simulink model "MCVT_Integration"  (R2020a)
settings: Solver=ode8  StartTime=0.0  StopTime=T_end  FixedStep=dt  RelTol=1e-3  EnableMultiTasking=on
values from neighbouring files (symbols this model uses):
  assumed_power_voltage = 1.25e2    (= 125)    [MCVT_Input_File.m]    (also in MCVT_Run_QuickCheck.m)
  battery_power_toggle = 1    [MCVT_Input_File.m]    (also in MCVT_Run_QuickCheck.m)
  battery_trickle_charge_param = 0.1    [MCVT_Input_File.m]    (also in MCVT_Run_QuickCheck.m)
  battery_trickle_leakage_param = 0.01    [MCVT_Input_File.m]    (also in MCVT_Run_QuickCheck.m)
  damage_case_id = 3    [MCVT_Input_File.m]    (also in MCVT_Run_QuickCheck.m = 1)
  dt = 1/fs    (= 0.001)    [MCVT_Run_QuickCheck.m]    (also in MCVT_Sim_Set.m)
  dt_2 = 1/fs_2    (= 0.001)    [MCVT_Run_QuickCheck.m]    (also in MCVT_Sim_Set.m)
  dt_3 = 1/fs_3    (= 0.001)    [MCVT_Run_QuickCheck.m]    (also in MCVT_Sim_Set.m)
  dt_5 = 1/fs_5    (= 0.005)    [MCVT_Run_QuickCheck.m]    (also in MCVT_Sim_Set.m)
  dt_8 = 1/fs_8    (= 0.05)    [MCVT_Run_QuickCheck.m]    (also in MCVT_Sim_Set.m)
  dt_9 = 1/fs_9    (= 0.001)    [MCVT_Run_QuickCheck.m]    (also in MCVT_Sim_Set.m)
  dust_met_duration = 3    [MCVT_Input_File.m]    (also in MCVT_Run_QuickCheck.m = 2)
  ECLSS_indoor_set_temperature = 298.15    [MCVT_Input_File.m]    (also in MCVT_Run_QuickCheck.m)
  energy_store_max_o = 150    [MCVT_Input_File.m]    (also in MCVT_Run_QuickCheck.m)
  energy_store_o = 120    [MCVT_Input_File.m]    (also in MCVT_Run_QuickCheck.m)
  fs = 1000    [MCVT_Run_QuickCheck.m]    (also in MCVT_Sim_Set.m)
  gain_charge_param = 1    [MCVT_Input_File.m]    (also in MCVT_Run_QuickCheck.m)
  ground_temperature = 373    [MCVT_Habitat_Design_Input_File.m]    (also in MCVT_Run_QuickCheck.m)
  I_max_charge = 800    [MCVT_Input_File.m]    (also in MCVT_Run_QuickCheck.m)
  I_max_discharge = 800    [MCVT_Input_File.m]    (also in MCVT_Run_QuickCheck.m)
  initial_pressure = 1e5    (= 100000)    [MCVT_Input_File.m]    (also in MCVT_Run_QuickCheck.m)
  initial_temperature = 295    [MCVT_Input_File.m]    (also in MCVT_Run_QuickCheck.m)
  interior_leak_rate = 0.00415    [MCVT_Input_File.m]    (also in MCVT_Run_QuickCheck.m)
  launch_dust_accum_start_time = 10    [MCVT_Input_File.m]    (also in MCVT_Run_QuickCheck.m = 2)
  launch_dust_accum_stop_time = 12    [MCVT_Input_File.m]    (also in MCVT_Run_QuickCheck.m = 3)
  meteorite_impact_time = 2    [MCVT_Input_File.m]    (also in MCVT_Run_QuickCheck.m = 1)
  nuclear_power_toggle = 1    [MCVT_Input_File.m]    (also in MCVT_Run_QuickCheck.m)
  print_interval = 30    [MCVT_Input_File.m]
  SOC_lower_thres = 0.5    [MCVT_Input_File.m]    (also in MCVT_Run_QuickCheck.m)
  SOC_upper_thres = 0.9    [MCVT_Input_File.m]    (also in MCVT_Run_QuickCheck.m)
  solar_power_toggle = 1    [MCVT_Input_File.m]    (also in MCVT_Run_QuickCheck.m)
  space_temperature = 4    [MCVT_Habitat_Design_Input_File.m]    (also in MCVT_Run_QuickCheck.m)
  Start_angle = 90    [MCVT_Input_File.m]    (also in MCVT_Run_QuickCheck.m)
undefined symbols (not defined in the file or in its folder): A_flow_restrictor, ACTIVITY_LOOKUP_TABLE, air, Aref, Bmap, Bmap2, Bmap3, comms, DiscIntNeverNeededParam, DmgSPL, dust_parameters, ECLSS_dust_damage_repair_time, ECLSS_heater_capacity, ECLSS_IPS_restrictor, ECLSS_IPS_setpointPressure, ECLSS_paint_damage_repair_time, ECLSS_radiatorLoop_discreteNumber, ECLSS_Vst, emptyActivity, expected_heat_add, FI, habitat, HIEM2SMM, HIEM2STM, HIEMDamageElements, impact_numbering, initial_SPLT_damage_index, input_air, input_Cond, input_CP, input_discretization, input_EV, input_Evap, input_radiatorLoop, K_ECLSS_dust_single, K_ECLSS_paint_single, K_Structure_damage_single, Kref, kW_to_W, meteoroidDustDep, mFRF, ndelays, nominalDustDep, noverlap, nuclear_toggle_gain, output_limits, panel_paint0, Ps0, reference_temperature, RepairTime_ECLSS_factor, Sensor_Location_SMM, SMM_T0, SMM_T1, SMM_T2, SMM_T3, SMM_T4, SMM_T4_0, SMM_T4_1, SMM_T4_2, solar_FDD_K ... (+73)
libraries: neural x350, simulink x39, xpcutilitieslib x7, xpcrtudplib x7, dspstat3 x1
(part 35 of 43)

== system MCVT_Integration/Sys2 - Structural System/Sys2_Structural_System/Fault Detection and Diagnosis (FDD) with Binary Classifer/Subsystem/Enabled Subsystem3/Pattern Recognition Neural Network1/Layer 3/Delays 1 ==
  "p{3}" : Inport  PortDimensions=20  SampleTime=0.001
      mask Display: e = 0.06;c1 = [120 230 180]/255;c2 = ([120 230 180]+0)/(256+128);c3 = ([120 230 180]+128)/(256+128);patch([0 1 1 0],[0 0 1 1],c1);patch([0 1 1 1-e 1-e 0+e],[0 0 1 1-e 0+e 0+e],c2);patch([0 0 1 1-e 0+e 0+e],[0 1 1 1-e 1-e 0+e],c3);plot([0 1 1 0 0],[0 0 1 1 0]);
  mux : Mux  Inputs=1
      mask Display: e = 0.06;c1 = [120 150 230]/255;c2 = ([120 150 230]+0)/383;c3 = ([120 150 230]+128)/383;patch([0 1 1 0],[0 0 1 1],c1);patch([0 1 1 1-e 1-e 0+e],[0 0 1 1-e 0+e 0+e],c2);patch([0 0 1 1-e 0+e 0+e],[0 1 1 1-e 1-e 0+e],c3);plot([0 1 1 0 0],[0 0 1 1 0]);disp('Mux')
  "pd{3,2}" : Outport
      mask Display: e = 0.06;c1 = [120 230 180]/255;c2 = ([120 230 180]+0)/(256+128);c3 = ([120 230 180]+128)/(256+128);patch([0 1 1 0],[0 0 1 1],c1);patch([0 1 1 1-e 1-e 0+e],[0 0 1 1-e 0+e 0+e],c2);patch([0 0 1 1-e 0+e 0+e],[0 1 1 1-e 1-e 0+e],c3);plot([0 1 1 0 0],[0 0 1 1 0]);
  wires:
  "p{3}"/1 -> mux/1
  mux/1 -> "pd{3,2}"/1

== system MCVT_Integration/Sys2 - Structural System/Sys2_Structural_System/Fault Detection and Diagnosis (FDD) with Binary Classifer/Subsystem/Enabled Subsystem3/Pattern Recognition Neural Network1/Layer 3/LW{3,2} ==
  "ad{3,2}" : Inport  PortDimensions=20  SampleTime=0.001
      mask Display: e = 0.06;c1 = [120 230 180]/255;c2 = ([120 230 180]+0)/(256+128);c3 = ([120 230 180]+128)/(256+128);patch([0 1 1 0],[0 0 1 1],c1);patch([0 1 1 1-e 1-e 0+e],[0 0 1 1-e 0+e 0+e],c2);patch([0 0 1 1-e 0+e 0+e],[0 1 1 1-e 1-e 0+e],c3);plot([0 1 1 0 0],[0 0 1 1 0]);
  "IW{3,2}(1,:)'" : Constant  Value=[-0.11418317317423039292112463272133027203381061553955078125;0.263496798393575704100300072241225279867649078369140625;0.312934394505613722170522805754444561898708343505859375;-0.450418381981469717612043268673005513846874237060546875;0.008234437834952347545769413272864767350256443023681640625;-0.462923222718843174749991931093973107635974884033203125;0.5964544880257796055644803345785476267337799072265625;-0.4783472265888535357447608475922606885433197021484375;-0.09036306949382867659270601734533556737005710601806640625;0.12308048153058916140967227192959398962557315826416015625;0.10743009166485244232358553517769905738532543182373046875;-0.481697721023931324513256413410999812185764312744140625;-0.47876976535250570510271472812746651470661163330078125;-0.711626168608504361401401183684356510639190673828125;0.225177975554882225939223872046568430960178375244140625;0.681899131620984366719540048507042229175567626953125;0.460863606080788301699868725336273200809955596923828125;-0.323125050863476370732740861058118753135204315185546875;-0.25662906632515347649103887306409887969493865966796875;0.480838665710967172461920426940196193754673004150390625]
      mask Display: e = 0.06;c1 = [120 150 230]/255;c2 = ([120 150 230]+0)/383;c3 = ([120 150 230]+128)/383;patch([0 1 1 0],[0 0 1 1],c1);patch([0 1 1 1-e 1-e 0+e],[0 0 1 1-e 0+e 0+e],c2);patch([0 0 1 1-e 0+e 0+e],[0 1 1 1-e 1-e 0+e],c3);plot([0 1 1 0 0],[0 0 1 1 0]);disp('weights');
  "IW{3,2}(10,:)'" : Constant  Value=[0.3861400843114013436974119031219743192195892333984375;0.3125216652004194628489130991511046886444091796875;-0.595476598820065650130572976195253431797027587890625;-0.037119852898883122505058196338723064400255680084228515625;0.189111040823012388489843260686029680073261260986328125;0.420303286434084044653758382992236874997615814208984375;-0.2676880868573281446742839762009680271148681640625;0.1753980171191553971699050862298463471233844757080078125;0.39560842290195019632648154583876021206378936767578125;0.69829375106497781899861365673132240772247314453125;0.50404441039708292038312720251269638538360595703125;0.252775726492291241687127012482960708439350128173828125;-0.1450315262218064005228512769463122822344303131103515625;0.0859287412860451904617065110869589261710643768310546875;0.133941298854357959680072553965146653354167938232421875;0.22618240937400890810948794751311652362346649169921875;0.379638748312004725704582597245462238788604736328125;-0.21496011361299871555985419036005623638629913330078125;-0.306329634482241985882211565694888122379779815673828125;0.302106512168412055796551385355996899306774139404296875]
      mask Display: e = 0.06;c1 = [120 150 230]/255;c2 = ([120 150 230]+0)/383;c3 = ([120 150 230]+128)/383;patch([0 1 1 0],[0 0 1 1],c1);patch([0 1 1 1-e 1-e 0+e],[0 0 1 1-e 0+e 0+e],c2);patch([0 0 1 1-e 0+e 0+e],[0 1 1 1-e 1-e 0+e],c3);plot([0 1 1 0 0],[0 0 1 1 0]);disp('weights');
  "IW{3,2}(2,:)'" : Constant  Value=[-0.163390928991248796098290085865301080048084259033203125;-0.0951760108873469234946895767279784195125102996826171875;-0.84593655133122869216322214924730360507965087890625;0.46899503026038946718045963280019350349903106689453125;-0.8490909409678917274533205272746272385120391845703125;-0.57545479794395382189264864791766740381717681884765625;-0.324175505751264803055988750202232040464878082275390625;0.364041861382856046791545168161974288523197174072265625;-0.2544481589174762969918219823739491403102874755859375;0.28990490623402187519985773178632371127605438232421875;-0.08466602028875479246661228671655408106744289398193359375;0.70073781097509135751266740044229663908481597900390625;-0.41615489274903116889703369452035985887050628662109375;0.2424679765837617073653831312185502611100673675537109375;-0.7464754937890354380414237311924807727336883544921875;-0.1596535806179091299128458558698184788227081298828125;0.7130628957660773981075408300966955721378326416015625;-0.43840295015644847875790901525761000812053680419921875;0.284476216686578020631515073546324856579303741455078125;0.447583082598702441945448526894324459135532379150390625]
      mask Display: e = 0.06;c1 = [120 150 230]/255;c2 = ([120 150 230]+0)/383;c3 = ([120 150 230]+128)/383;patch([0 1 1 0],[0 0 1 1],c1);patch([0 1 1 1-e 1-e 0+e],[0 0 1 1-e 0+e 0+e],c2);patch([0 0 1 1-e 0+e 0+e],[0 1 1 1-e 1-e 0+e],c3);plot([0 1 1 0 0],[0 0 1 1 0]);disp('weights');
  "IW{3,2}(3,:)'" : Constant  Value=[0.0098602709082808159823940741262049414217472076416015625;-0.40815757787930284639088540643570013344287872314453125;0.12336924569621048053402745381390559487044811248779296875;-0.6190044151957840856681514196679927408695220947265625;-0.564487784933820790911340736784040927886962890625;0.54853651299544214925418827988323755562305450439453125;-1.258259749472828925576095571159385144710540771484375;-0.77927996917740427473830777671537362039089202880859375;0.16813033094256812916000853874720633029937744140625;0.10729529541650513480011142064540763385593891143798828125;0.262661701867050567660299975614179857075214385986328125;-0.0733259301165432308966529717508819885551929473876953125;1.22466710827414804185764296562410891056060791015625;0.271802237285291459212288600610918365418910980224609375;-0.71351834644215295089253459082101471722126007080078125;-0.74660981018017091859206857407116331160068511962890625;0.5983682870257907016053877669037319719791412353515625;-0.01351833734841678680282939239987172186374664306640625;0.051967795942245952056648405914529575966298580169677734375;0.1434699887749444624507333401197683997452259063720703125]
      mask Display: e = 0.06;c1 = [120 150 230]/255;c2 = ([120 150 230]+0)/383;c3 = ([120 150 230]+128)/383;patch([0 1 1 0],[0 0 1 1],c1);patch([0 1 1 1-e 1-e 0+e],[0 0 1 1-e 0+e 0+e],c2);patch([0 0 1 1-e 0+e 0+e],[0 1 1 1-e 1-e 0+e],c3);plot([0 1 1 0 0],[0 0 1 1 0]);disp('weights');
  "IW{3,2}(4,:)'" : Constant  Value=[-0.039394049345288499608397358997535775415599346160888671875;-0.320240693032457890421227375554735772311687469482421875;0.203468281254232497001765977984177879989147186279296875;0.138427932331368575002983334343298338353633880615234375;0.488490423588747113559094259471748955547809600830078125;0.276252854526421176561967740781255997717380523681640625;0.104006018020532609025252668288885615766048431396484375;0.51873376787390912401320974822738207876682281494140625;0.48405322090183655614481494922074489295482635498046875;-0.06304979425881369892170624780192156322300434112548828125;-0.392143975943062106370717856407281942665576934814453125;-0.75218739963867531361785268018138594925403594970703125;0.71739453453784574055163147932034917175769805908203125;-0.443935510913416486733495958105777390301227569580078125;0.78728550848033951314874911986407823860645294189453125;-0.120688688580087466473145241252495907247066497802734375;-0.53856777060676896962121418255264870822429656982421875;0.3444710945141284952342175529338419437408447265625;0.25306929741619488272164062436786480247974395751953125;0.331976039764520780561696255972492508590221405029296875]
      mask Display: e = 0.06;c1 = [120 150 230]/255;c2 = ([120 150 230]+0)/383;c3 = ([120 150 230]+128)/383;patch([0 1 1 0],[0 0 1 1],c1);patch([0 1 1 1-e 1-e 0+e],[0 0 1 1-e 0+e 0+e],c2);patch([0 0 1 1-e 0+e 0+e],[0 1 1 1-e 1-e 0+e],c3);plot([0 1 1 0 0],[0 0 1 1 0]);disp('weights');
  "IW{3,2}(5,:)'" : Constant  Value=[0.323360971356433768431060116199660114943981170654296875;-0.281984417075820503750804846276878379285335540771484375;-0.289282489355113858753298927695141173899173736572265625;0.4782575856980688655539779574610292911529541015625;-0.642831136730397378897805538144893944263458251953125;1.0145734120541420875127869294374249875545501708984375;0.2595396344103828578653292424860410392284393310546875;0.66796656307939361596481830929405987262725830078125;-0.46150839887887240564623425598256289958953857421875;0.306587228188199223399834636438754387199878692626953125;-0.94949409796797734628626130870543420314788818359375;-0.03140176831624992559444109474497963674366474151611328125;0.295677475903499298315324494978995062410831451416015625;-0.5493980409988299395962485505151562392711639404296875;0.403008193931628400097366693444200791418552398681640625;0.9733051449238032670763232090394012629985809326171875;-0.8510176648196960957903911548783071339130401611328125;-0.2207833887391404126265825880182092078030109405517578125;-0.278018396739915463200532030896283686161041259765625;0.393882424770033490180054513984941877424716949462890625]
      mask Display: e = 0.06;c1 = [120 150 230]/255;c2 = ([120 150 230]+0)/383;c3 = ([120 150 230]+128)/383;patch([0 1 1 0],[0 0 1 1],c1);patch([0 1 1 1-e 1-e 0+e],[0 0 1 1-e 0+e 0+e],c2);patch([0 0 1 1-e 0+e 0+e],[0 1 1 1-e 1-e 0+e],c3);plot([0 1 1 0 0],[0 0 1 1 0]);disp('weights');
  "IW{3,2}(6,:)'" : Constant  Value=[-0.3606616604959353988846260108402930200099945068359375;-0.1704191509480204036464101591263897716999053955078125;0.150526929813042997796657118669827468693256378173828125;0.1472315563777921998411812865015235729515552520751953125;-0.1278164823918129522350994875523610971868038177490234375;-1.046809064148225498769306796020828187465667724609375;0.582795720158978181046904865070246160030364990234375;0.75223224770795427129854715531109832227230072021484375;0.33523115012585902316999408867559395730495452880859375;-0.338211234277506933576518122208653949201107025146484375;-0.390605230615673482130745242102420888841152191162109375;-0.287165688343693215056617873415234498679637908935546875;-0.70909893212667751694056050837389193475246429443359375;-0.7038433128654613124552952285739593207836151123046875;-0.86812992371547170744605637082713656127452850341796875;-0.1746870548125196986877227800505352206528186798095703125;-0.410264069210815929267255341983400285243988037109375;-0.48871178689720362608994719266775064170360565185546875;-0.05532824759820657856135284191623213700950145721435546875;0.05704494121705543097089474713357049040496349334716796875]
      mask Display: e = 0.06;c1 = [120 150 230]/255;c2 = ([120 150 230]+0)/383;c3 = ([120 150 230]+128)/383;patch([0 1 1 0],[0 0 1 1],c1);patch([0 1 1 1-e 1-e 0+e],[0 0 1 1-e 0+e 0+e],c2);patch([0 0 1 1-e 0+e 0+e],[0 1 1 1-e 1-e 0+e],c3);plot([0 1 1 0 0],[0 0 1 1 0]);disp('weights');
  "IW{3,2}(7,:)'" : Constant  Value=[0.11984386036742382397601858201596769504249095916748046875;0.347080609380766791360883871675468981266021728515625;0.0917862807948270631452913903558510355651378631591796875;-0.288490135071363951180245521754841320216655731201171875;0.63114059686687029593343822853057645261287689208984375;-0.5584052675142625599136181335779838263988494873046875;0.53125277720215191568087220730376429855823516845703125;-0.3716338061699360206802111861179582774639129638671875;-0.05721538948189093531926374680551816709339618682861328125;-0.71575407318186801131076890669646672904491424560546875;-0.08369009494839028950874393331105238758027553558349609375;-0.215724270354954394957047725256416015326976776123046875;1.2396797863463160371821913940948434174060821533203125;0.47994944326806088241710313013754785060882568359375;-0.00717360076498131486733011996648201602511107921600341796875;0.22905992881372438940701385945430956780910491943359375;-0.976685274095676181360659029451198875904083251953125;-0.180881724828344025990389809521730057895183563232421875;-0.294597694843611368487046320296940393745899200439453125;0.04199206334362116932457098528175265528261661529541015625]
      mask Display: e = 0.06;c1 = [120 150 230]/255;c2 = ([120 150 230]+0)/383;c3 = ([120 150 230]+128)/383;patch([0 1 1 0],[0 0 1 1],c1);patch([0 1 1 1-e 1-e 0+e],[0 0 1 1-e 0+e 0+e],c2);patch([0 0 1 1-e 0+e 0+e],[0 1 1 1-e 1-e 0+e],c3);plot([0 1 1 0 0],[0 0 1 1 0]);disp('weights');
  "IW{3,2}(8,:)'" : Constant  Value=[-0.21342261365704862097203431403613649308681488037109375;0.35624143718627421240086050602258183062076568603515625;0.326618800376075990588020658833556808531284332275390625;0.06597532960682862823720284950468339957296848297119140625;-0.17461288648652029120711404175381176173686981201171875;-0.263005374932626601047758185814018361270427703857421875;-0.049151473469004801353232636529355659149587154388427734375;0.766371952396857292200138545013032853603363037109375;-0.1355212253420195744890719424802227877080440521240234375;-0.56439442500416614478808696730993688106536865234375;-0.71372482706963469301086888663121499121189117431640625;0.12115721620020099280612413394919713027775287628173828125;-0.254628175010240986875231783415074460208415985107421875;0.2614167249770866785496536977007053792476654052734375;0.57295880892063089095955774610047228634357452392578125;0.54430137291944247213137941798777319490909576416015625;0.59699489786736725793758751024142839014530181884765625;-0.085575093688173564654420033548376522958278656005859375;0.038297841824984357739225515615544281899929046630859375;0.372723897650229674116673095340956933796405792236328125]
      mask Display: e = 0.06;c1 = [120 150 230]/255;c2 = ([120 150 230]+0)/383;c3 = ([120 150 230]+128)/383;patch([0 1 1 0],[0 0 1 1],c1);patch([0 1 1 1-e 1-e 0+e],[0 0 1 1-e 0+e 0+e],c2);patch([0 0 1 1-e 0+e 0+e],[0 1 1 1-e 1-e 0+e],c3);plot([0 1 1 0 0],[0 0 1 1 0]);disp('weights');
  "IW{3,2}(9,:)'" : Constant  Value=[-0.1914423406104446556685871883018990047276020050048828125;-0.393308930523766420161990708947996608912944793701171875;1.2635378199134894572353005059994757175445556640625;1.059262594219074760104604138177819550037384033203125;-0.73547758546770169996165122938691638410091400146484375;-1.7230018270653120193713903063326142728328704833984375;0.193088656561395455302232448957511223852634429931640625;-0.1291887260637646084315832695210701785981655120849609375;0.08111166291770897929236383561146794818341732025146484375;0.07141734494336980654249913413877948187291622161865234375;0.93639924412624753191636273186304606497287750244140625;0.369705966288347009918169305819901637732982635498046875;-0.5856153767568719192837534137652255594730377197265625;-0.449137029161876977223499807223561219871044158935546875;0.65634022147763559917876818872173316776752471923828125;0.68121028217221801792646829198929481208324432373046875;1.0442129309195211650518331225612200796604156494140625;-0.531155452284312712407654544222168624401092529296875;0.435977640337065930342674846542649902403354644775390625;0.7223516834459691171588247016188688576221466064453125]
      mask Display: e = 0.06;c1 = [120 150 230]/255;c2 = ([120 150 230]+0)/383;c3 = ([120 150 230]+128)/383;patch([0 1 1 0],[0 0 1 1],c1);patch([0 1 1 1-e 1-e 0+e],[0 0 1 1-e 0+e 0+e],c2);patch([0 0 1 1-e 0+e 0+e],[0 1 1 1-e 1-e 0+e],c3);plot([0 1 1 0 0],[0 0 1 1 0]);disp('weights');
  Mux : Mux  Inputs=10
      mask Display: e = 0.06;c1 = [120 150 230]/255;c2 = ([120 150 230]+0)/383;c3 = ([120 150 230]+128)/383;patch([0 1 1 0],[0 0 1 1],c1);patch([0 1 1 1-e 1-e 0+e],[0 0 1 1-e 0+e 0+e],c2);patch([0 0 1 1-e 0+e 0+e],[0 1 1 1-e 1-e 0+e],c3);plot([0 1 1 0 0],[0 0 1 1 0]);disp('Mux');
  dotprod{1..10} (10 alike) : neural/Weight Functions/dotprod
  "lz{3,2}" : Outport
      mask Display: e = 0.06;c1 = [120 230 180]/255;c2 = ([120 230 180]+0)/(256+128);c3 = ([120 230 180]+128)/(256+128);patch([0 1 1 0],[0 0 1 1],c1);patch([0 1 1 1-e 1-e 0+e],[0 0 1 1-e 0+e 0+e],c2);patch([0 0 1 1-e 0+e 0+e],[0 1 1 1-e 1-e 0+e],c3);plot([0 1 1 0 0],[0 0 1 1 0]);
  wires:
  "IW{3,2}(1,:)'"/1 -> dotprod1/1
  "ad{3,2}"/1 -> dotprod1/2, dotprod2/2, dotprod3/2, dotprod4/2, dotprod5/2, dotprod6/2, dotprod7/2, dotprod8/2, dotprod9/2, dotprod10/2
  dotprod1/1 -> Mux/1
  "IW{3,2}(2,:)'"/1 -> dotprod2/1
  dotprod2/1 -> Mux/2
  "IW{3,2}(3,:)'"/1 -> dotprod3/1
  dotprod3/1 -> Mux/3
  "IW{3,2}(4,:)'"/1 -> dotprod4/1
  dotprod4/1 -> Mux/4
  "IW{3,2}(5,:)'"/1 -> dotprod5/1
  dotprod5/1 -> Mux/5
  "IW{3,2}(6,:)'"/1 -> dotprod6/1
  dotprod6/1 -> Mux/6
  "IW{3,2}(7,:)'"/1 -> dotprod7/1
  dotprod7/1 -> Mux/7
  "IW{3,2}(8,:)'"/1 -> dotprod8/1
  dotprod8/1 -> Mux/8
  "IW{3,2}(9,:)'"/1 -> dotprod9/1
  dotprod9/1 -> Mux/9
  "IW{3,2}(10,:)'"/1 -> dotprod10/1
  dotprod10/1 -> Mux/10
  Mux/1 -> "lz{3,2}"/1

== system MCVT_Integration/Sys2 - Structural System/Sys2_Structural_System/Fault Detection and Diagnosis (FDD) with Binary Classifer/Subsystem/Enabled Subsystem3/Pattern Recognition Neural Network1/Layer 4/Delays 1 ==
  "p{4}" : Inport  PortDimensions=10  SampleTime=0.001
      mask Display: e = 0.06;c1 = [120 230 180]/255;c2 = ([120 230 180]+0)/(256+128);c3 = ([120 230 180]+128)/(256+128);patch([0 1 1 0],[0 0 1 1],c1);patch([0 1 1 1-e 1-e 0+e],[0 0 1 1-e 0+e 0+e],c2);patch([0 0 1 1-e 0+e 0+e],[0 1 1 1-e 1-e 0+e],c3);plot([0 1 1 0 0],[0 0 1 1 0]);
  mux : Mux  Inputs=1
      mask Display: e = 0.06;c1 = [120 150 230]/255;c2 = ([120 150 230]+0)/383;c3 = ([120 150 230]+128)/383;patch([0 1 1 0],[0 0 1 1],c1);patch([0 1 1 1-e 1-e 0+e],[0 0 1 1-e 0+e 0+e],c2);patch([0 0 1 1-e 0+e 0+e],[0 1 1 1-e 1-e 0+e],c3);plot([0 1 1 0 0],[0 0 1 1 0]);disp('Mux')
  "pd{4,3}" : Outport
      mask Display: e = 0.06;c1 = [120 230 180]/255;c2 = ([120 230 180]+0)/(256+128);c3 = ([120 230 180]+128)/(256+128);patch([0 1 1 0],[0 0 1 1],c1);patch([0 1 1 1-e 1-e 0+e],[0 0 1 1-e 0+e 0+e],c2);patch([0 0 1 1-e 0+e 0+e],[0 1 1 1-e 1-e 0+e],c3);plot([0 1 1 0 0],[0 0 1 1 0]);
  wires:
  "p{4}"/1 -> mux/1
  mux/1 -> "pd{4,3}"/1

== system MCVT_Integration/Sys2 - Structural System/Sys2_Structural_System/Fault Detection and Diagnosis (FDD) with Binary Classifer/Subsystem/Enabled Subsystem3/Pattern Recognition Neural Network1/Layer 4/LW{4,3} ==
  "ad{4,3}" : Inport  PortDimensions=10  SampleTime=0.001
      mask Display: e = 0.06;c1 = [120 230 180]/255;c2 = ([120 230 180]+0)/(256+128);c3 = ([120 230 180]+128)/(256+128);patch([0 1 1 0],[0 0 1 1],c1);patch([0 1 1 1-e 1-e 0+e],[0 0 1 1-e 0+e 0+e],c2);patch([0 0 1 1-e 0+e 0+e],[0 1 1 1-e 1-e 0+e],c3);plot([0 1 1 0 0],[0 0 1 1 0]);
  "IW{4,3}(1,:)'" : Constant  Value=[-0.51604720910410739609375241343514062464237213134765625;-1.7366033358789458862503352065687067806720733642578125;1.3466704923413341798976716745528392493724822998046875;0.828798695930853757118939029169268906116485595703125;0.773836988737240272229200854781083762645721435546875;-1.7885580142512098600349190746783278882503509521484375;1.6156847911129046391209840294322930276393890380859375;-0.7352668019450157022021130615030415356159210205078125;-0.69193546212086121105500069461413659155368804931640625;-0.28924703412926555667894490397884510457515716552734375]
      mask Display: e = 0.06;c1 = [120 150 230]/255;c2 = ([120 150 230]+0)/383;c3 = ([120 150 230]+128)/383;patch([0 1 1 0],[0 0 1 1],c1);patch([0 1 1 1-e 1-e 0+e],[0 0 1 1-e 0+e 0+e],c2);patch([0 0 1 1-e 0+e 0+e],[0 1 1 1-e 1-e 0+e],c3);plot([0 1 1 0 0],[0 0 1 1 0]);disp('weights');
  "IW{4,3}(2,:)'" : Constant  Value=[-0.0202306625362932111789060485307345516048371791839599609375;0.22411300199437411695413402412668801844120025634765625;-1.8347562896376488073002519740839488804340362548828125;0.99359854707041483923291025348589755594730377197265625;3.1641844715110654107093068887479603290557861328125;0.5631442586802963834458068959065712988376617431640625;-0.369739837029956885938730692942044697701930999755859375;0.67101973187238339590976465842686593532562255859375;-0.93642832144661325433077081470401026308536529541015625;-0.014970032274003695904607269540065317414700984954833984375]
      mask Display: e = 0.06;c1 = [120 150 230]/255;c2 = ([120 150 230]+0)/383;c3 = ([120 150 230]+128)/383;patch([0 1 1 0],[0 0 1 1],c1);patch([0 1 1 1-e 1-e 0+e],[0 0 1 1-e 0+e 0+e],c2);patch([0 0 1 1-e 0+e 0+e],[0 1 1 1-e 1-e 0+e],c3);plot([0 1 1 0 0],[0 0 1 1 0]);disp('weights');
  "IW{4,3}(3,:)'" : Constant  Value=[-0.272318370748756366506171389119117520749568939208984375;1.3106242908829701665496259010978974401950836181640625;1.312630158079725273267968077561818063259124755859375;-0.21086989260161981274421805210295133292675018310546875;-1.259158188825396873511408557533286511898040771484375;0.04911585360940884381619753185077570378780364990234375;-1.209163369118716246930489432998001575469970703125;-0.518358873752605031626217169105075299739837646484375;1.970145207538365639265975914895534515380859375;-0.759002889593023866154908319003880023956298828125]
      mask Display: e = 0.06;c1 = [120 150 230]/255;c2 = ([120 150 230]+0)/383;c3 = ([120 150 230]+128)/383;patch([0 1 1 0],[0 0 1 1],c1);patch([0 1 1 1-e 1-e 0+e],[0 0 1 1-e 0+e 0+e],c2);patch([0 0 1 1-e 0+e 0+e],[0 1 1 1-e 1-e 0+e],c3);plot([0 1 1 0 0],[0 0 1 1 0]);disp('weights');
  "IW{4,3}(4,:)'" : Constant  Value=[-1.202951395306457893497054101317189633846282958984375;1.65808938734872146625320965540595352649688720703125;0.9837936780281901771871844175620935857295989990234375;-1.0065905119791309640930876412312500178813934326171875;0.4548559843469706098773031044402159750461578369140625;0.1814816976308144536034205884789116680622100830078125;0.1334806823514721740853161691120476461946964263916015625;-0.056173163191362028567876762963351211510598659515380859375;-2.9090275687083870792548623285256326198577880859375;0.80815879831503190100505662485375069081783294677734375]
      mask Display: e = 0.06;c1 = [120 150 230]/255;c2 = ([120 150 230]+0)/383;c3 = ([120 150 230]+128)/383;patch([0 1 1 0],[0 0 1 1],c1);patch([0 1 1 1-e 1-e 0+e],[0 0 1 1-e 0+e 0+e],c2);patch([0 0 1 1-e 0+e 0+e],[0 1 1 1-e 1-e 0+e],c3);plot([0 1 1 0 0],[0 0 1 1 0]);disp('weights');
  "IW{4,3}(5,:)'" : Constant  Value=[-0.335059031903964188447986316532478667795658111572265625;-0.70944732098641238859926261284272186458110809326171875;-2.07230031086661714567753733717836439609527587890625;0.01433875060803528785069449469347091508097946643829345703125;-0.89513067392367406949205133059876970946788787841796875;1.788542097246339945826321127242408692836761474609375;0.95917862069667647606507898672134615480899810791015625;0.44762254473427898204818120575509965419769287109375;1.2224214755361841877601136729936115443706512451171875;-0.681303755031184454793447002884931862354278564453125]
      mask Display: e = 0.06;c1 = [120 150 230]/255;c2 = ([120 150 230]+0)/383;c3 = ([120 150 230]+128)/383;patch([0 1 1 0],[0 0 1 1],c1);patch([0 1 1 1-e 1-e 0+e],[0 0 1 1-e 0+e 0+e],c2);patch([0 0 1 1-e 0+e 0+e],[0 1 1 1-e 1-e 0+e],c3);plot([0 1 1 0 0],[0 0 1 1 0]);disp('weights');
  Mux : Mux  Inputs=5
      mask Display: e = 0.06;c1 = [120 150 230]/255;c2 = ([120 150 230]+0)/383;c3 = ([120 150 230]+128)/383;patch([0 1 1 0],[0 0 1 1],c1);patch([0 1 1 1-e 1-e 0+e],[0 0 1 1-e 0+e 0+e],c2);patch([0 0 1 1-e 0+e 0+e],[0 1 1 1-e 1-e 0+e],c3);plot([0 1 1 0 0],[0 0 1 1 0]);disp('Mux');
  dotprod{1..5} (5 alike) : neural/Weight Functions/dotprod
  "lz{4,3}" : Outport
      mask Display: e = 0.06;c1 = [120 230 180]/255;c2 = ([120 230 180]+0)/(256+128);c3 = ([120 230 180]+128)/(256+128);patch([0 1 1 0],[0 0 1 1],c1);patch([0 1 1 1-e 1-e 0+e],[0 0 1 1-e 0+e 0+e],c2);patch([0 0 1 1-e 0+e 0+e],[0 1 1 1-e 1-e 0+e],c3);plot([0 1 1 0 0],[0 0 1 1 0]);
  wires:
  "IW{4,3}(1,:)'"/1 -> dotprod1/1
  "ad{4,3}"/1 -> dotprod1/2, dotprod2/2, dotprod3/2, dotprod4/2, dotprod5/2
  dotprod1/1 -> Mux/1
  "IW{4,3}(2,:)'"/1 -> dotprod2/1
  dotprod2/1 -> Mux/2
  "IW{4,3}(3,:)'"/1 -> dotprod3/1
  dotprod3/1 -> Mux/3
  "IW{4,3}(4,:)'"/1 -> dotprod4/1
  dotprod4/1 -> Mux/4
  "IW{4,3}(5,:)'"/1 -> dotprod5/1
  dotprod5/1 -> Mux/5
  Mux/1 -> "lz{4,3}"/1

== system MCVT_Integration/Sys3 - Power System/Power Generation Subsystem1/Nuclear Power Generation/SP-100 Power Generation/	Power Generation/Nominal Power/Sensor Models/Cold Junction Model ==
  Cold Junction Temperature : Constant
  Kelvin to Celcuis : Bias  Bias=-273.15
  Out1 : Outport
  wires:
  Cold Junction Temperature/1 -> Kelvin to Celcuis/1
  Kelvin to Celcuis/1 -> Out1/1

== system MCVT_Integration/Sys3 - Power System/Power Generation Subsystem1/Nuclear Power Generation/SP-100 Power Generation/	Power Generation/Nominal Power/Sensor Models/Cold Junction Temperature Sensing Model ==
  note: Switch Control
      | 1 - Switch ON
      | 2 - Switch OFF
  In1 : Inport
  " Resistance Temperature Detector" : Subsystem
  " Switch ON/OFF Control Number" : Constant
  Celcuis to Kelvin : Bias  Bias=273.15
  Out1 : Outport
  wires:
  In1/1 -> " Resistance Temperature Detector"/1(Physical Interdependency - IN)
  " Switch ON/OFF Control Number"/1 -> " Resistance Temperature Detector"/2(Switch Control)
  Celcuis to Kelvin/1 -> Out1/1
  " Resistance Temperature Detector"/2(Measured Temperature) -> Celcuis to Kelvin/1  "Measured Temperature"

== system MCVT_Integration/Sys3 - Power System/Power Generation Subsystem1/Nuclear Power Generation/SP-100 Power Generation/	Power Generation/Nominal Power/Sensor Models/Cold Junction Temperature Sensing Model1 ==
  note: Switch Control
      | 1 - Switch ON
      | 2 - Switch OFF
  In1 : Inport
  " Resistance Temperature Detector" : Subsystem
  " Switch ON/OFF Control Number" : Constant
  Celcuis to Kelvin : Bias  Bias=273.15
  Out1 : Outport
  wires:
  In1/1 -> " Resistance Temperature Detector"/1(Physical Interdependency - IN)
  " Switch ON/OFF Control Number"/1 -> " Resistance Temperature Detector"/2(Switch Control)
  Celcuis to Kelvin/1 -> Out1/1
  " Resistance Temperature Detector"/2(Measured Temperature) -> Celcuis to Kelvin/1  "Measured Temperature"

== system MCVT_Integration/Sys3 - Power System/Power Generation Subsystem1/Nuclear Power Generation/SP-100 Power Generation/	Power Generation/Nominal Power/Sensor Models/Cold Junction Temperature Sensing Model2 ==
  note: Switch Control
      | 1 - Switch ON
      | 2 - Switch OFF
  In1 : Inport
  " Resistance Temperature Detector" : Subsystem
  " Switch ON/OFF Control Number" : Constant
  Celcuis to Kelvin : Bias  Bias=273.15
  Out1 : Outport
  wires:
  " Resistance Temperature Detector"/2(Measured Temperature) -> Celcuis to Kelvin/1  "Measured Temperature"
  Celcuis to Kelvin/1 -> Out1/1
  " Switch ON/OFF Control Number"/1 -> " Resistance Temperature Detector"/2(Switch Control)
  In1/1 -> " Resistance Temperature Detector"/1(Physical Interdependency - IN)

== system MCVT_Integration/Sys3 - Power System/Power Generation Subsystem1/Nuclear Power Generation/SP-100 Power Generation/	Power Generation/Nominal Power/Sensor Models/Cold Junction Temperature Sensing Model3 ==
  note: Switch Control
      | 1 - Switch ON
      | 2 - Switch OFF
  In1 : Inport
  " Resistance Temperature Detector" : Subsystem
  " Switch ON/OFF Control Number" : Constant
  Celcuis to Kelvin : Bias  Bias=273.15
  Out1 : Outport
  wires:
  In1/1 -> " Resistance Temperature Detector"/1(Physical Interdependency - IN)
  " Switch ON/OFF Control Number"/1 -> " Resistance Temperature Detector"/2(Switch Control)
  Celcuis to Kelvin/1 -> Out1/1
  " Resistance Temperature Detector"/2(Measured Temperature) -> Celcuis to Kelvin/1  "Measured Temperature"

== system MCVT_Integration/Sys3 - Power System/Power Generation Subsystem1/Nuclear Power Generation/SP-100 Power Generation/	Power Generation/Nominal Power/Sensor Models/Cold Junction Temperature Sensing Model4 ==
  note: Switch Control
      | 1 - Switch ON
      | 2 - Switch OFF
  In1 : Inport
  " Resistance Temperature Detector" : Subsystem
  " Switch ON/OFF Control Number" : Constant
  Celcuis to Kelvin : Bias  Bias=273.15
  Out1 : Outport
  wires:
  " Resistance Temperature Detector"/2(Measured Temperature) -> Celcuis to Kelvin/1  "Measured Temperature"
  Celcuis to Kelvin/1 -> Out1/1
  " Switch ON/OFF Control Number"/1 -> " Resistance Temperature Detector"/2(Switch Control)
  In1/1 -> " Resistance Temperature Detector"/1(Physical Interdependency - IN)

== system MCVT_Integration/Sys3 - Power System/Power Generation Subsystem1/Nuclear Power Generation/SP-100 Power Generation/	Power Generation/Nominal Power/Sensor Models/Power Meter Stub ==
  In1 : Inport
  Out1 : Outport
  wires:
  In1/1 -> Out1/1

== system MCVT_Integration/Sys3 - Power System/Power Generation Subsystem1/Nuclear Power Generation/SP-100 Power Generation/	Power Generation/Nominal Power/Sensor Models/Thermocouple Mode Stub ==
  In1 : Inport
  In2 : Inport  Port=2
  "MAX31856 Precision Thermocouple to Digital Converter with Linearization " : Subsystem
  Omega XMO S-type Thermocouple : Subsystem
  Estimated Tf : Outport
  Cyber : Outport  Port=2
  wires:
  "MAX31856 Precision Thermocouple to Digital Converter with Linearization "/2(Cyber Output) -> Cyber/1
  In2/1 -> "MAX31856 Precision Thermocouple to Digital Converter with Linearization "/2(External Cold Junction Measurement)
  "MAX31856 Precision Thermocouple to Digital Converter with Linearization "/1(Estimated Process Temperature) -> Estimated Tf/1
  In1/1 -> Omega XMO S-type Thermocouple/1(Actual Temperature)
  Omega XMO S-type Thermocouple/1(microvolts) -> "MAX31856 Precision Thermocouple to Digital Converter with Linearization "/1(Thermocouple Measurement)

== system MCVT_Integration/Sys3 - Power System/Power Generation Subsystem1/Nuclear Power Generation/SP-100 Power Generation/	Power Generation/Nominal Power/Sensor Models/Thermocouple Model Stub ==
  In1 : Inport
  In2 : Inport  Port=2
  "MAX31856 Precision Thermocouple to Digital Converter with Linearization " : Subsystem
  Omega XMO S-type Thermocouple : Subsystem
  Estimated Tb1 : Outport
  Cyber : Outport  Port=2
  wires:
  Omega XMO S-type Thermocouple/1(microvolts) -> "MAX31856 Precision Thermocouple to Digital Converter with Linearization "/1(Thermocouple Measurement)
  In2/1 -> "MAX31856 Precision Thermocouple to Digital Converter with Linearization "/2(External Cold Junction Measurement)
  "MAX31856 Precision Thermocouple to Digital Converter with Linearization "/2(Cyber Output) -> Cyber/1
  In1/1 -> Omega XMO S-type Thermocouple/1(Actual Temperature)
  "MAX31856 Precision Thermocouple to Digital Converter with Linearization "/1(Estimated Process Temperature) -> Estimated Tb1/1

== system MCVT_Integration/Sys3 - Power System/Power Generation Subsystem1/Nuclear Power Generation/SP-100 Power Generation/	Power Generation/Nominal Power/Sensor Models/Thermocouple Model2 Stub ==
  In1 : Inport
  In2 : Inport  Port=2
  "MAX31856 Precision Thermocouple to Digital Converter with Linearization " : Subsystem
  Omega XMO S-type Thermocouple : Subsystem
  Estimated Tb2 : Outport
  Cyber : Outport  Port=2
  wires:
  Omega XMO S-type Thermocouple/1(microvolts) -> "MAX31856 Precision Thermocouple to Digital Converter with Linearization "/1(Thermocouple Measurement)
  In2/1 -> "MAX31856 Precision Thermocouple to Digital Converter with Linearization "/2(External Cold Junction Measurement)
  "MAX31856 Precision Thermocouple to Digital Converter with Linearization "/2(Cyber Output) -> Cyber/1
  In1/1 -> Omega XMO S-type Thermocouple/1(Actual Temperature)
  "MAX31856 Precision Thermocouple to Digital Converter with Linearization "/1(Estimated Process Temperature) -> Estimated Tb2/1

== system MCVT_Integration/Sys3 - Power System/Power Generation Subsystem1/Nuclear Power Generation/SP-100 Power Generation/	Power Generation/Nominal Power/Sensor Models/Thermocouple Model3 Stub ==
  In1 : Inport
  In2 : Inport  Port=2
  "MAX31856 Precision Thermocouple to Digital Converter with Linearization " : Subsystem
  Omega XMO S-type Thermocouple : Subsystem
  Estimated Tc1 : Outport
  Cyber : Outport  Port=2
  wires:
  Omega XMO S-type Thermocouple/1(microvolts) -> "MAX31856 Precision Thermocouple to Digital Converter with Linearization "/1(Thermocouple Measurement)
  In2/1 -> "MAX31856 Precision Thermocouple to Digital Converter with Linearization "/2(External Cold Junction Measurement)
  "MAX31856 Precision Thermocouple to Digital Converter with Linearization "/2(Cyber Output) -> Cyber/1
  In1/1 -> Omega XMO S-type Thermocouple/1(Actual Temperature)
  "MAX31856 Precision Thermocouple to Digital Converter with Linearization "/1(Estimated Process Temperature) -> Estimated Tc1/1

== system MCVT_Integration/Sys3 - Power System/Power Generation Subsystem1/Nuclear Power Generation/SP-100 Power Generation/	Power Generation/Nominal Power/Sensor Models/Thermocouple Model4 Stub ==
  In1 : Inport
  In2 : Inport  Port=2
  "MAX31856 Precision Thermocouple to Digital Converter with Linearization " : Subsystem
  Omega XMO S-type Thermocouple : Subsystem
  Estimated Tc2 : Outport
  Cyber : Outport  Port=2
  wires:
  Omega XMO S-type Thermocouple/1(microvolts) -> "MAX31856 Precision Thermocouple to Digital Converter with Linearization "/1(Thermocouple Measurement)
  In2/1 -> "MAX31856 Precision Thermocouple to Digital Converter with Linearization "/2(External Cold Junction Measurement)
  "MAX31856 Precision Thermocouple to Digital Converter with Linearization "/2(Cyber Output) -> Cyber/1
  In1/1 -> Omega XMO S-type Thermocouple/1(Actual Temperature)
  "MAX31856 Precision Thermocouple to Digital Converter with Linearization "/1(Estimated Process Temperature) -> Estimated Tc2/1

== system MCVT_Integration/Sys3 - Power System/Power Generation Subsystem1/Nuclear Power Generation/SP-100 Power Generation/	Power Generation/Nominal Power/Sensor Models/block ==
  y_process_scale : Inport
  Power Out : Inport  Port=2
  Tc1_lower : Inport  Port=3
  Bus Creator : BusCreator  Inputs=6
  MATLAB Function2 : MATLAB Function  TreatAsAtomicUnit=on
  Bus : Outport
  wires:
  MATLAB Function2/3(y_Tb2) -> Bus Creator/3  "Tb2 Primary Coolant Outlet Temperature_nom"
  Tc1_lower/1 -> Bus Creator/6  "Tc1 Secondary Coolant Inlet Temperature_nom"
  MATLAB Function2/1(y_Tf) -> Bus Creator/1  "TF Fuel Temperature_nom"
  Power Out/1 -> Bus Creator/5  "power_out_nom"
  MATLAB Function2/2(y_Tb1) -> Bus Creator/2  "Tb1 Primary Coolant Inlet Temperature_nom"
  y_process_scale/1 -> MATLAB Function2/1(y_process_scale_lower)
  MATLAB Function2/4(y_Tc2) -> Bus Creator/4  "Tc2 Secondary Coolant Outlet Temperature_nom"
  Bus Creator/1 -> Bus/1

== system MCVT_Integration/Sys3 - Power System/Power Generation Subsystem1/Nuclear Power Generation/SP-100 Power Generation/	Power Generation/Sensor Models/Cold Junction Temperature Sensing Model/ Resistance Temperature Detector ==
  Physical Interdependency - IN : Inport
  Switch Control : Inport  Port=2
  R = U/I : Gain  Gain=1/0.001
  R vs T : MATLAB Function  TreatAsAtomicUnit=on
  RTD with Self-heat Error : Subsystem
  RTD without Self-heat Error : Subsystem
  Self-heat Calculation : Subsystem
  Switch ON/OFF Control Block : Subsystem
  T(Celcuis) vs R : MATLAB Function  TreatAsAtomicUnit=on
  Read Temperature : Outport  VectorParamsAs1DForOutWhenUnconnected=off
  Measured Temperature : Outport  Port=2  VectorParamsAs1DForOutWhenUnconnected=off
  wires:
  T(Celcuis) vs R/1(R) -> RTD without Self-heat Error/1(Resistance Change without Error), Self-heat Calculation/2(Resistance Change without Error)
  R vs T/1(T) -> Measured Temperature/1
  RTD with Self-heat Error/1(Voltage Output with Error) -> R = U/I/1
  Self-heat Calculation/1(Resistance Change with Error) -> RTD with Self-heat Error/1(Resistance Change with Error)
  R = U/I/1 -> R vs T/1(R)
  RTD without Self-heat Error/1(Current in Circuit) -> Self-heat Calculation/3(Current in Circuit)
  Switch ON/OFF Control Block/1(Out1) -> T(Celcuis) vs R/1(T), Self-heat Calculation/1(Real Temperature)
  Switch Control/1 -> Switch ON/OFF Control Block/1(In1)
  Physical Interdependency - IN/1 -> Read Temperature/1, Switch ON/OFF Control Block/2(In2)

== system MCVT_Integration/Sys3 - Power System/Power Generation Subsystem1/Nuclear Power Generation/SP-100 Power Generation/	Power Generation/Sensor Models/Cold Junction Temperature Sensing Model1/ Resistance Temperature Detector ==
  Physical Interdependency - IN : Inport
  Switch Control : Inport  Port=2
  R = U/I : Gain  Gain=1/0.001
  R vs T : MATLAB Function  TreatAsAtomicUnit=on
  RTD with Self-heat Error : Subsystem
  RTD without Self-heat Error : Subsystem
  Self-heat Calculation : Subsystem
  Switch ON/OFF Control Block : Subsystem
  T(Celcuis) vs R : MATLAB Function  TreatAsAtomicUnit=on
  Temperature Output with Error : Scope
  Read Temperature : Outport  VectorParamsAs1DForOutWhenUnconnected=off
  Measured Temperature : Outport  Port=2  VectorParamsAs1DForOutWhenUnconnected=off
  wires:
  T(Celcuis) vs R/1(R) -> RTD without Self-heat Error/1(Resistance Change without Error), Self-heat Calculation/2(Resistance Change without Error)
  R vs T/1(T) -> Measured Temperature/1, Temperature Output with Error/1
  RTD with Self-heat Error/1(Voltage Output with Error) -> R = U/I/1
  Self-heat Calculation/1(Resistance Change with Error) -> RTD with Self-heat Error/1(Resistance Change with Error)
  R = U/I/1 -> R vs T/1(R)
  RTD without Self-heat Error/1(Current in Circuit) -> Self-heat Calculation/3(Current in Circuit)
  Switch ON/OFF Control Block/1(Out1) -> T(Celcuis) vs R/1(T), Self-heat Calculation/1(Real Temperature)
  Switch Control/1 -> Switch ON/OFF Control Block/1(In1)
  Physical Interdependency - IN/1 -> Read Temperature/1, Switch ON/OFF Control Block/2(In2)

== system MCVT_Integration/Sys3 - Power System/Power Generation Subsystem1/Nuclear Power Generation/SP-100 Power Generation/	Power Generation/Sensor Models/Cold Junction Temperature Sensing Model2/ Resistance Temperature Detector ==
  Physical Interdependency - IN : Inport
  Switch Control : Inport  Port=2
  R = U/I : Gain  Gain=1/0.001
  R vs T : MATLAB Function  TreatAsAtomicUnit=on
  RTD with Self-heat Error : Subsystem
  RTD without Self-heat Error : Subsystem
  Self-heat Calculation : Subsystem
  Switch ON/OFF Control Block : Subsystem
  T(Celcuis) vs R : MATLAB Function  TreatAsAtomicUnit=on
  Temperature Output with Error : Scope
  Read Temperature : Outport  VectorParamsAs1DForOutWhenUnconnected=off
  Measured Temperature : Outport  Port=2  VectorParamsAs1DForOutWhenUnconnected=off
  wires:
  Physical Interdependency - IN/1 -> Switch ON/OFF Control Block/2(In2), Read Temperature/1
  Switch Control/1 -> Switch ON/OFF Control Block/1(In1)
  Switch ON/OFF Control Block/1(Out1) -> Self-heat Calculation/1(Real Temperature), T(Celcuis) vs R/1(T)
  RTD without Self-heat Error/1(Current in Circuit) -> Self-heat Calculation/3(Current in Circuit)
  R = U/I/1 -> R vs T/1(R)
  Self-heat Calculation/1(Resistance Change with Error) -> RTD with Self-heat Error/1(Resistance Change with Error)
  RTD with Self-heat Error/1(Voltage Output with Error) -> R = U/I/1
  R vs T/1(T) -> Temperature Output with Error/1, Measured Temperature/1
  T(Celcuis) vs R/1(R) -> Self-heat Calculation/2(Resistance Change without Error), RTD without Self-heat Error/1(Resistance Change without Error)

== system MCVT_Integration/Sys3 - Power System/Power Generation Subsystem1/Nuclear Power Generation/SP-100 Power Generation/	Power Generation/Sensor Models/Cold Junction Temperature Sensing Model3/ Resistance Temperature Detector ==
  Physical Interdependency - IN : Inport
  Switch Control : Inport  Port=2
  R = U/I : Gain  Gain=1/0.001
  R vs T : MATLAB Function  TreatAsAtomicUnit=on
  RTD with Self-heat Error : Subsystem
  RTD without Self-heat Error : Subsystem
  Self-heat Calculation : Subsystem
  Switch ON/OFF Control Block : Subsystem
  T(Celcuis) vs R : MATLAB Function  TreatAsAtomicUnit=on
  Temperature Output with Error : Scope
  Read Temperature : Outport  VectorParamsAs1DForOutWhenUnconnected=off
  Measured Temperature : Outport  Port=2  VectorParamsAs1DForOutWhenUnconnected=off
  wires:
  T(Celcuis) vs R/1(R) -> RTD without Self-heat Error/1(Resistance Change without Error), Self-heat Calculation/2(Resistance Change without Error)
  R vs T/1(T) -> Measured Temperature/1, Temperature Output with Error/1
  RTD with Self-heat Error/1(Voltage Output with Error) -> R = U/I/1
  Self-heat Calculation/1(Resistance Change with Error) -> RTD with Self-heat Error/1(Resistance Change with Error)
  R = U/I/1 -> R vs T/1(R)
  RTD without Self-heat Error/1(Current in Circuit) -> Self-heat Calculation/3(Current in Circuit)
  Switch ON/OFF Control Block/1(Out1) -> T(Celcuis) vs R/1(T), Self-heat Calculation/1(Real Temperature)
  Switch Control/1 -> Switch ON/OFF Control Block/1(In1)
  Physical Interdependency - IN/1 -> Read Temperature/1, Switch ON/OFF Control Block/2(In2)

== system MCVT_Integration/Sys3 - Power System/Power Generation Subsystem1/Nuclear Power Generation/SP-100 Power Generation/	Power Generation/Sensor Models/Cold Junction Temperature Sensing Model4/ Resistance Temperature Detector ==
  Physical Interdependency - IN : Inport
  Switch Control : Inport  Port=2
  R = U/I : Gain  Gain=1/0.001
  R vs T : MATLAB Function  TreatAsAtomicUnit=on
  RTD with Self-heat Error : Subsystem
  RTD without Self-heat Error : Subsystem
  Self-heat Calculation : Subsystem
  Switch ON/OFF Control Block : Subsystem
  T(Celcuis) vs R : MATLAB Function  TreatAsAtomicUnit=on
  Temperature Output with Error : Scope
  Read Temperature : Outport  VectorParamsAs1DForOutWhenUnconnected=off
  Measured Temperature : Outport  Port=2  VectorParamsAs1DForOutWhenUnconnected=off
  wires:
  Physical Interdependency - IN/1 -> Switch ON/OFF Control Block/2(In2), Read Temperature/1
  Switch Control/1 -> Switch ON/OFF Control Block/1(In1)
  Switch ON/OFF Control Block/1(Out1) -> Self-heat Calculation/1(Real Temperature), T(Celcuis) vs R/1(T)
  RTD without Self-heat Error/1(Current in Circuit) -> Self-heat Calculation/3(Current in Circuit)
  R = U/I/1 -> R vs T/1(R)
  Self-heat Calculation/1(Resistance Change with Error) -> RTD with Self-heat Error/1(Resistance Change with Error)
  RTD with Self-heat Error/1(Voltage Output with Error) -> R = U/I/1
  R vs T/1(T) -> Temperature Output with Error/1, Measured Temperature/1
  T(Celcuis) vs R/1(R) -> Self-heat Calculation/2(Resistance Change without Error), RTD without Self-heat Error/1(Resistance Change without Error)

== system MCVT_Integration/Sys3 - Power System/Power Generation Subsystem1/Nuclear Power Generation/SP-100 Power Generation/	Power Generation/Sensor Models/Thermocouple Mode Stub/MAX31856 Precision Thermocouple to Digital Converter with Linearization  ==
  Thermocouple Measurement : Inport
  External Cold Junction Measurement : Inport  Port=2
  Celcuis to Kelvin : Bias  Bias=273.15
  Thermocouple conditioning 7 : Lookup_n-D  NumberOfTableDimensions=1  BreakpointsForDimension1=TypeS_Volts  BeginIndexSearchUsingPreviousIndexResult=on  Table=TypeS_degC  DiagnosticForOutOfRangeInput=Warning  InputSameDT=on
      settings: AttributesFormatString=%<bp1> to\n%<tableData>
  Estimated Process Temperature : Outport
  Cyber Output : Outport  Port=2
  wires:
  Thermocouple conditioning 7/1 -> Celcuis to Kelvin/1
  Thermocouple Measurement/1 -> Thermocouple conditioning 7/1
  Celcuis to Kelvin/1 -> Estimated Process Temperature/1

== system MCVT_Integration/Sys3 - Power System/Power Generation Subsystem1/Nuclear Power Generation/SP-100 Power Generation/	Power Generation/Sensor Models/Thermocouple Mode Stub/Omega XMO S-type Thermocouple ==
  Actual Temperature : Inport
  Kelvin to Celcuis : Bias  Bias=-273.15
  Thermocouple model 7 : Lookup_n-D  NumberOfTableDimensions=1  BreakpointsForDimension1=TypeS_degC  BeginIndexSearchUsingPreviousIndexResult=on  Table=TypeS_Volts  DiagnosticForOutOfRangeInput=Warning  InputSameDT=on
      settings: AttributesFormatString=%<bp1> to\n%<tableData>
  microvolts : Outport
  wires:
  Actual Temperature/1 -> Kelvin to Celcuis/1
  Kelvin to Celcuis/1 -> Thermocouple model 7/1
  Thermocouple model 7/1 -> microvolts/1

== system MCVT_Integration/Sys3 - Power System/Power Generation Subsystem1/Nuclear Power Generation/SP-100 Power Generation/	Power Generation/Sensor Models/Thermocouple Model Stub/MAX31856 Precision Thermocouple to Digital Converter with Linearization  ==
  Thermocouple Measurement : Inport
  External Cold Junction Measurement : Inport  Port=2
  Celcuis to Kelvin : Bias  Bias=273.15
  Thermocouple conditioning 7 : Lookup_n-D  NumberOfTableDimensions=1  BreakpointsForDimension1=TypeS_Volts  BeginIndexSearchUsingPreviousIndexResult=on  Table=TypeS_degC  DiagnosticForOutOfRangeInput=Warning  InputSameDT=on
      settings: AttributesFormatString=%<bp1> to\n%<tableData>
  Estimated Process Temperature : Outport
  Cyber Output : Outport  Port=2
  wires:
  Thermocouple conditioning 7/1 -> Celcuis to Kelvin/1
  Thermocouple Measurement/1 -> Thermocouple conditioning 7/1
  Celcuis to Kelvin/1 -> Estimated Process Temperature/1
